simulink model "x64_32f_024i_gbe"  (Simulink 7.4) (continued)
== system x64_32f_024i_gbe == (continued)
  gpio_a_oen : xps_library/gpio  Tag=xps:gpio  SystemSampleTime=-1  io_group=ROACH:gpioa_oe_n  io_dir=out  arith_type=Boolean  bitwidth=1  bin_pt=0  bit_index=0  sample_period=1  use_single_ended=off  use_ddr=off  reg_iob=on  reg_clk_phase=0  termination=Pulldown
  led1 : xps_library/gpio  Tag=xps:gpio  SystemSampleTime=-1  io_group=ROACH:gpiob  io_dir=out  arith_type=Boolean  bitwidth=1  bin_pt=0  bit_index=1  sample_period=1  use_single_ended=off  use_ddr=off  reg_iob=on  reg_clk_phase=0  termination=Pulldown
  led2 : xps_library/gpio  Tag=xps:gpio  SystemSampleTime=-1  io_group=ROACH:gpiob  io_dir=out  arith_type=Boolean  bitwidth=1  bin_pt=0  bit_index=2  sample_period=1  use_single_ended=off  use_ddr=off  reg_iob=on  reg_clk_phase=0  termination=Pulldown
  led3 : xps_library/gpio  Tag=xps:gpio  SystemSampleTime=-1  io_group=ROACH:gpiob  io_dir=out  arith_type=Boolean  bitwidth=1  bin_pt=0  bit_index=3  sample_period=1  use_single_ended=off  use_ddr=off  reg_iob=on  reg_clk_phase=0  termination=Pulldown
  negedge : casper_library_misc/negedge  Tag=Rising Edge Detector  SystemSampleTime=-1
  never{,1..3,5..6,10} (7 alike) : xbsIndex_r4/Constant  arith_type=Boolean  const=0  n_bits=16  bin_pt=14  explicit_period=on  period=1  equ=P=C  opselect=C  inp2=PCIN>>17  opr=+  inp1=P  carry=CIN  dbl_ovrd=off  block_type=constant
      settings: block_version=11.4
  never4 : xbsIndex_r4/Constant  arith_type=Unsigned  const=0  n_bits=16  bin_pt=0  explicit_period=on  period=1  equ=P=C  opselect=C  inp2=PCIN>>17  opr=+  inp1=P  carry=CIN  dbl_ovrd=off  block_type=constant
      settings: block_version=11.4
  never8 : xbsIndex_r4/Constant  arith_type=Unsigned  const=0  n_bits=32  bin_pt=0  explicit_period=on  period=1  equ=P=C  opselect=C  inp2=PCIN>>17  opr=+  inp1=P  carry=CIN  dbl_ovrd=off  block_type=constant
      settings: block_version=11.4
  not_zero : Subsystem
  pfb_fir_mux1 : Subsystem  AncestorBlock=casper_library_pfbs/pfb_fir_mux  mask(pfb_fir_mux){PFBSize=11, mux_inputs=2, TotalTaps=4, WindowType=hamming, n_inputs=0, MakeBiplex=1, BitWidthIn=12, BitWidthOut=18, CoeffBitWidth=18, CoeffDistMem=0, add_latency=1, mult_latency=2, bram_latency=3, quantization=Round  (unbiased: +/- Inf), fwidth=1, specify_mult=off, mult_spec=[2                                      2]}
      mask prompts: PFBSize "Size of PFB: (2^? pnts)"; mux_inputs "Signals per Input (2^?)"; TotalTaps "Total Number of Taps:"; WindowType "Windowing Function:"; n_inputs "Number of Simultaneous Inputs: (2^?)"; MakeBiplex "Make Biplex"; BitWidthIn "Input Bitwidth:"; BitWidthOut "Output Bitwidth:"; CoeffBitWidth "Coefficient Bitwidth:"; CoeffDistMem "Use Distributed Memory for Coeffs"; add_latency "Add Latency"; mult_latency "Mult Latency"; bram_latency "BRAM Latency"; quantization "Quantization Behavior"; fwidth "Bin Width Scaling (normal=1)"; specify_mult "Specify multiplier use (behavioral if not specified)"; mult_spec "Multiplier specification (0=core, 1=embedded, 2=behavioural) (left=1st tap)"
      mask init:
      | pfb_fir_mux_init(gcb, ...
      |     'PFBSize', PFBSize, ...
      |     'mux_inputs', mux_inputs, ...
      |     'TotalTaps', TotalTaps, ...
      |     'WindowType', WindowType, ...
      |     'n_inputs', n_inputs, ...
      |     'MakeBiplex', MakeBiplex, ...
      |     'BitWidthIn', BitWidthIn, ...
      |     'BitWidthOut', BitWidthOut, ...
      |     'CoeffBitWidth', CoeffBitWidth, ...
      |     'CoeffDistMem', CoeffDistMem, ...
      |     'add_latency', add_latency, ...
      |     'mult_latency', mult_latency, ...
      |     'bram_latency', bram_latency, ...
      |     'quantization', quantization, ...
      |     'fwidth', fwidth,...
      |     'specify_mult', specify_mult,...
      |     'mult_spec', mult_spec);
      settings: AttributesFormatString=taps=4, add_latency=1
      mask Help: eval('xlWeb(''http://casper.berkeley.edu/wiki/Pfb_fir'')')
      mask StyleString: edit,edit,edit,popup(bartlett|barthannwin|blackman|blackmanharris|bohamwin|chebwin|flattopwin|gausswin|hamming|hann|kaiser|nuttallwin|parzenwin|rectwin|tukeywin|triang|userwindow),edit,edit,edit,edit,edit,edit,edit,edit,edit,popup(Truncate|Round  (unbiased: +/- Inf)|Round  (unbiased: Even Values)),edit,checkbox,edit
      mask CallbackString:
      | ||%num_taps = eval(get_param(gcb, 'TotalTaps'));
      | %old_vec = eval(get_param(gcb, 'mult_spec'));
      | 
      | %vec = 2.*ones(1,num_taps);
      | %old_size = length(old_vec);
      | 
      | %if(old_size <= num_taps),
      | %   new_size = old_size;
      | %else,
      | %    new_size = num_taps;
      | %end
      | 
      | %for n = 1:new_size, vec(n) = old_vec(n); end
      | %set_param(gcb, 'mult_spec', mat2str(vec));||||||||||||%num_taps = eval(get_param(gcb, 'TotalTaps'));
      | %old_vec = eval(get_param(gcb, 'mult_spec'));
      | 
      | %vec = 2.*ones(1,num_taps);
      | %old_size = length(old_vec);
      | 
      | %if(old_size <= num_taps),
      | %    new_size = old_size;
      | %else,
      | %    new_size = num_taps;
      | %end
      | 
      | %for n = 1:new_size, vec(n) = old_vec(n); end
      | %set_param(gcb, 'mult_spec', mat2str(vec));|%specify_mult = get_param(gcb,'specify_mult');
      | %if( strcmp(specify_mult,'off')),
      | %    set_param(gcb, 'MaskEnables', {'on','on','on','on','on','on','on','on','on','on','on','on','on','on','on','off'});
      | %else
      | %    set_param(gcb, 'MaskEnables', {'on','on','on','on','on','on','on','on','on','on','on','on','on','on','on','on'});
      | %end
      | 
      | |%specify_mult = get_param(gcb,'specify_mult');
      | %if( strcmp(specify_mult,'off')),
      | %    set_param(gcb, 'MaskEnables', {'on','on','on','on','on','on','on','on','on','on','on','on','on','on','on','off'});
      | %else
      | %    set_param(gcb, 'MaskEnables', {'on','on','on','on','on','on','on','on','on','on','on','on','on','on','on','on'});
      | %end
  pfb_fir_mux2 : Subsystem  AncestorBlock=casper_library_pfbs/pfb_fir_mux  mask(pfb_fir_mux){PFBSize=11, mux_inputs=2, TotalTaps=4, WindowType=hamming, n_inputs=0, MakeBiplex=1, BitWidthIn=12, BitWidthOut=18, CoeffBitWidth=18, CoeffDistMem=0, add_latency=1, mult_latency=2, bram_latency=3, quantization=Round  (unbiased: +/- Inf), fwidth=1, specify_mult=off, mult_spec=[2                                      2]}
      mask prompts: PFBSize "Size of PFB: (2^? pnts)"; mux_inputs "Signals per Input (2^?)"; TotalTaps "Total Number of Taps:"; WindowType "Windowing Function:"; n_inputs "Number of Simultaneous Inputs: (2^?)"; MakeBiplex "Make Biplex"; BitWidthIn "Input Bitwidth:"; BitWidthOut "Output Bitwidth:"; CoeffBitWidth "Coefficient Bitwidth:"; CoeffDistMem "Use Distributed Memory for Coeffs"; add_latency "Add Latency"; mult_latency "Mult Latency"; bram_latency "BRAM Latency"; quantization "Quantization Behavior"; fwidth "Bin Width Scaling (normal=1)"; specify_mult "Specify multiplier use (behavioral if not specified)"; mult_spec "Multiplier specification (0=core, 1=embedded, 2=behavioural) (left=1st tap)"
      mask init: same as x64_32f_024i_gbe/pfb_fir_mux1
      settings: AttributesFormatString=taps=4, add_latency=1
      mask Help: eval('xlWeb(''http://casper.berkeley.edu/wiki/Pfb_fir'')')
      mask StyleString: edit,edit,edit,popup(bartlett|barthannwin|blackman|blackmanharris|bohamwin|chebwin|flattopwin|gausswin|hamming|hann|kaiser|nuttallwin|parzenwin|rectwin|tukeywin|triang|userwindow),edit,edit,edit,edit,edit,edit,edit,edit,edit,popup(Truncate|Round  (unbiased: +/- Inf)|Round  (unbiased: Even Values)),edit,checkbox,edit
      mask CallbackString:
      | ||%num_taps = eval(get_param(gcb, 'TotalTaps'));
      | %old_vec = eval(get_param(gcb, 'mult_spec'));
      | 
      | %vec = 2.*ones(1,num_taps);
      | %old_size = length(old_vec);
      | 
      | %if(old_size <= num_taps),
      | %   new_size = old_size;
      | %else,
      | %    new_size = num_taps;
      | %end
      | 
      | %for n = 1:new_size, vec(n) = old_vec(n); end
      | %set_param(gcb, 'mult_spec', mat2str(vec));||||||||||||%num_taps = eval(get_param(gcb, 'TotalTaps'));
      | %old_vec = eval(get_param(gcb, 'mult_spec'));
      | 
      | %vec = 2.*ones(1,num_taps);
      | %old_size = length(old_vec);
      | 
      | %if(old_size <= num_taps),
      | %    new_size = old_size;
      | %else,
      | %    new_size = num_taps;
      | %end
      | 
      | %for n = 1:new_size, vec(n) = old_vec(n); end
      | %set_param(gcb, 'mult_spec', mat2str(vec));|%specify_mult = get_param(gcb,'specify_mult');
      | %if( strcmp(specify_mult,'off')),
      | %    set_param(gcb, 'MaskEnables', {'on','on','on','on','on','on','on','on','on','on','on','on','on','on','on','off'});
      | %else
      | %    set_param(gcb, 'MaskEnables', {'on','on','on','on','on','on','on','on','on','on','on','on','on','on','on','on'});
      | %end
      | 
      | |%specify_mult = get_param(gcb,'specify_mult');
      | %if( strcmp(specify_mult,'off')),
      | %    set_param(gcb, 'MaskEnables', {'on','on','on','on','on','on','on','on','on','on','on','on','on','on','on','off'});
      | %else
      | %    set_param(gcb, 'MaskEnables', {'on','on','on','on','on','on','on','on','on','on','on','on','on','on','on','on'});
      | %end
  phase_EQ0 : Subsystem  mask{N=11-1+5-2-1, bram_lat=2, mult_lat=3, add_lat=2}
      mask prompts: N "number of coefficients (2^?)"; bram_lat "bram latency"; mult_lat "multiplier latency"; add_lat "adder latency"
      mask init:
      | set_param([gcb, '/coeff_bram'], 'addr_width', num2str(N));
  phase_EQ1 : Subsystem  mask{N=11-1+5-2-1, bram_lat=2, mult_lat=3, add_lat=2}
      mask prompts: N "number of coefficients (2^?)"; bram_lat "bram latency"; mult_lat "multiplier latency"; add_lat "adder latency"
      mask init:
      | set_param([gcb, '/coeff_bram'], 'addr_width', num2str(N));
  phase_EQ2 : Subsystem  mask{N=11-1+5-2-1, bram_lat=2, mult_lat=3, add_lat=2}
      mask prompts: N "number of coefficients (2^?)"; bram_lat "bram latency"; mult_lat "multiplier latency"; add_lat "adder latency"
      mask init:
      | set_param([gcb, '/coeff_bram'], 'addr_width', num2str(N));
  phase_EQ3 : Subsystem  mask{N=11-1+5-2-1, bram_lat=2, mult_lat=3, add_lat=2}
      mask prompts: N "number of coefficients (2^?)"; bram_lat "bram latency"; mult_lat "multiplier latency"; add_lat "adder latency"
      mask init:
      | set_param([gcb, '/coeff_bram'], 'addr_width', num2str(N));
  pipeline : Subsystem  AncestorBlock=casper_library/Delays/pipeline  mask(pipeline){latency=1}
      mask help:
      | An explicitly laid-out delay line to avoid mapping to SRL16s
      | for use in pipelining to help achieve timing closure.
      mask init:
      | pipeline_init(gcb, 'latency', latency);
      mask Display:
      | port_label('input', 1, 'd');
      | port_label('output', 1, 'q');
      | disp(['Z^-', num2str(latency)]);
  pipeline102 : Subsystem  AncestorBlock=casper_library/Delays/pipeline  mask(pipeline){latency=1}
      mask help:
      | An explicitly laid-out delay line to avoid mapping to SRL16s
      | for use in pipelining to help achieve timing closure.
      mask init:
      | pipeline_init(gcb, 'latency', latency);
      mask Display:
      | port_label('input', 1, 'd');
      | port_label('output', 1, 'q');
      | disp(['Z^-', num2str(latency)]);
  pipeline3 : Subsystem  AncestorBlock=casper_library/Delays/pipeline  mask(pipeline){latency=1}
      mask help:
      | An explicitly laid-out delay line to avoid mapping to SRL16s
      | for use in pipelining to help achieve timing closure.
      mask init:
      | pipeline_init(gcb, 'latency', latency);
      mask Display:
      | port_label('input', 1, 'd');
      | port_label('output', 1, 'q');
      | disp(['Z^-', num2str(latency)]);
  qdr_transpose1 : Subsystem  AncestorBlock=casper_library/Reorder/qdr_transpose  mask{idepth=(11-1), odepth=10, which_qdr=qdr1}
      mask prompts: idepth "Input blocksize (2^n)"; odepth "Output blocksize (2^n)"; which_qdr "QDR Chip"
      mask init:
      | new_map = tostring([[0:2^odepth-1]*2 [0:2^odepth-1]*2 + 1]);
      | set_param([gcb, '/reorder'], 'map', new_map);
      | set_param([gcb, '/qdr'], 'which_qdr', which_qdr);
      mask StyleString: edit,edit,popup(qdr0|qdr1)
  quant : Subsystem
  quant1 : Subsystem
  quant2 : Subsystem
  quant3 : Subsystem
  quant4 : Subsystem
  quant5 : Subsystem
  quant6 : Subsystem
  quant7 : Subsystem
  reorder : Subsystem  AncestorBlock=casper_library/Reorder/reorder  mask(reorder){map=getfield( getfield( get_param( gcb,'UserData' ), 'parameters'),'map'), n_inputs=1, bram_latency=2, map_latency=0, double_buffer=0, bram_map=off}
      mask prompts: map "Output Order:"; n_inputs "Number of inputs"; bram_latency "BRAM Latency"; map_latency "Map Latency"; double_buffer "Double Buffer"; bram_map "Use BlockRAM for Map"
      mask init:
      | reorder_init(gcb, ...
      |     'map', map, ...
      |     'n_inputs', n_inputs, ...
      |     'bram_latency', bram_latency, ...
      |     'map_latency', map_latency, ...
      |     'double_buffer', double_buffer, ...
      |     'bram_map', bram_map);
      settings: AttributesFormatString=order=10
      mask Help: eval('xlWeb(''http://casper.berkeley.edu/wiki/Reorder'')')
  reorder_one_ant_a_time1 : Subsystem  mask{spec_chans=11-1, block_size=7}
      mask prompts: spec_chans "frequency channels? (2^?) (NFFT/2 for real FFTs)"; block_size "block size (2^?)"
      mask init:
      | partial_reorder = [0:2:2^block_size - 1]
      | 
      | reorder = []
      | for n = [0:1]
      |     reorder = [reorder, [partial_reorder]+n];
      | end
      | 
      | reorder
      | 
      | set_param([gcb, '/reorder'], 'map', tostring(reorder));
  revision_control : Subsystem  AncestorBlock=casper_library_misc/revision_control  mask(revision_control){rev_major=24, rev_minor=1, error_on_dirty=off, error_on_norcs=off}
      mask prompts: rev_major "Major revision number"; rev_minor "Minor revision number"; error_on_dirty "Error on out-of-date"; error_on_norcs "Error on no revision control"
      mask init:
      | revision_control_init( gcb, ...
      |   'rev_major', rev_major, ...
      |   'rev_minor', rev_minor, ...
      |   'error_on_dirty', error_on_dirty, ...
      |   'error_on_norcs', error_on_norcs);
  ri_to_c{,1..3} (4 alike) : casper_library_misc/ri_to_c  SystemSampleTime=-1
  seng_oob_signal : Subsystem
  seng_packetizer : Subsystem  mask{nwrd_bits=5, ant_bits=4}
      mask prompts: nwrd_bits "Payload length: (in 64bit words) (2^n)"; ant_bits "Number of windows per channel(2^?)"
      mask help: Package 32 bit data into 64 bits and identify data as coming from antenna 0 or 1
  sim_sync : ox_lib/sim_sync  SystemSampleTime=-1  delay=9
  sim_sync1 : ox_lib/sim_sync  SystemSampleTime=-1  delay=10
  snap_ctrl : xps_library/software register  Tag=xps:sw_reg  SystemSampleTime=-1  io_dir=From Processor  arith_type=Unsigned  bitwidth=32  bin_pt=0  sample_period=1  gw_name=x64_32f_024i_gbe_snap_ctrl_user_data_out
  status : Subsystem  Tag=xps:sw_reg  mask{io_dir=To Processor, arith_type=Unsigned, bitwidth=32, bin_pt=0, sample_period=1, latency=0}
      mask prompts: io_dir "I/O direction"; arith_type "Data Type"; bitwidth "Data bitwidth"; bin_pt "Data binary point"; sample_period "Sample period"
      mask init:
      | gw_name = clear_name(gcb);
      | config.source = str2func('software_register_init');
      | config.toplevel = gcb;
      | xBlock( config, {io_dir, arith_type, bitwidth, bin_pt, ... 
      |     sample_period, gw_name, latency});
      mask StyleString: popup(From Processor|To Processor),popup(Unsigned|Signed  (2's comp)),edit,edit,edit,edit
  sync_gen : Subsystem  mask{period=10010*2^20}
  sync_gpio : xps_library/gpio  Tag=xps:gpio  SystemSampleTime=-1  io_group=ROACH:gpioa  io_dir=in  arith_type=Boolean  bitwidth=1  bin_pt=0  bit_index=7  sample_period=1  use_single_ended=off  use_ddr=off  reg_iob=on  reg_clk_phase=0  termination=Pullup
  sync_rate_change : Subsystem  mask{bits=10+3}
      mask prompts: bits "New sync rate: 2^? clocks?"
      mask help: Increases the sync pulses. For use in, for example, a snap block, where the snap can be triggered every 2^NFFT clocks, but the system sync may come much less frequently.
  sync_rate_change1 : Subsystem  mask{bits=10+3}
      mask prompts: bits "New sync rate: 2^? clocks?"
      mask help: Increases the sync pulses. For use in, for example, a snap block, where the snap can be triggered every 2^NFFT clocks, but the system sync may come much less frequently.
  sync_rate_change2 : Subsystem  mask{bits=10+3}
      mask prompts: bits "New sync rate: 2^? clocks?"
      mask help: Increases the sync pulses. For use in, for example, a snap block, where the snap can be triggered every 2^NFFT clocks, but the system sync may come much less frequently.
  sync_rate_change3 : Subsystem  mask{bits=10+3}
      mask prompts: bits "New sync rate: 2^? clocks?"
      mask help: Increases the sync pulses. For use in, for example, a snap block, where the snap can be triggered every 2^NFFT clocks, but the system sync may come much less frequently.
  sync_rate_change4 : Subsystem  mask{bits=10+3}
      mask prompts: bits "New sync rate: 2^? clocks?"
      mask help: Increases the sync pulses. For use in, for example, a snap block, where the snap can be triggered every 2^NFFT clocks, but the system sync may come much less frequently.
  ten_Gbe_v2 : xps_library/ten_Gbe_v2  Tag=xps:tengbe_v2  SystemSampleTime=-1  flavour=cx4  slot=1  port_r1=2  port_r2_cx4=0  port_r2_sfpp=0  rx_dist_ram=on  large_frames=on  show_param=on  pre_emph_r2=0.74  pre_emph=3  post_emph_r2=0.18  rxeqmix_r2=7  swing_r2=940  swing=800  fab_en=on  fab_mac=hex2dec('123456780000')  fab_ip=192*(2^24) + 168*(2^16) + 5*(2^8) + 20*(2^0)  fab_udp=10000  fab_gate=1  cpu_rx_en=on  cpu_tx_en=on
  uncram : Subsystem  AncestorBlock=gavrt_library/uncram  mask(uncram){num_slice=8, slice_width=1, bin_pt=0, arith_type=0}
      mask prompts: num_slice "Number of slices"; slice_width "Slice Width"; bin_pt "Output Binary Point"; arith_type "Output Arithmetic Type (0=Unsigned, 1=Signed)"
      mask help: Takes a concatenated input and slices it up into even pieces and reinterprets them as signed fixed point numbers with a given binary point.
      mask init:
      | uncram_init(gcb, ...
      |     'num_slice', num_slice, ...
      |     'slice_width', slice_width, ...
      |     'bin_pt', bin_pt, ...
      |     'arith_type', arith_type);
      settings: AttributesFormatString=UFix_1_0
  uncram1 : Subsystem  AncestorBlock=gavrt_library/uncram  mask(uncram){num_slice=8, slice_width=1, bin_pt=0, arith_type=0}
      mask prompts: num_slice "Number of slices"; slice_width "Slice Width"; bin_pt "Output Binary Point"; arith_type "Output Arithmetic Type (0=Unsigned, 1=Signed)"
      mask help: Takes a concatenated input and slices it up into even pieces and reinterprets them as signed fixed point numbers with a given binary point.
      mask init:
      | uncram_init(gcb, ...
      |     'num_slice', num_slice, ...
      |     'slice_width', slice_width, ...
      |     'bin_pt', bin_pt, ...
      |     'arith_type', arith_type);
      settings: AttributesFormatString=UFix_1_0
  x64_adc : Subsystem  Tag=xps:x64_adc  AncestorBlock=xps_library/x64_adc  mask(x64_adc){adc_clk_rate=40, spi=off, ctrl_gpio=GPIO_B}
      mask prompts: adc_clk_rate "ADC clock rate (MHz)"; spi "Include SPI interface?"; ctrl_gpio "GPIO bank to use for control signals?"
      mask help: The ADC block converts analog inputs to digital outputs. Every clock cycle, the inputs are sampled and digitized to 8 bit binary point numbers in the range of [-1, 1) and are then output by the adc.
      mask init:
      | x64_adc_mask(gcb, 'spi', spi);
      mask Help: eval('xlWeb([getenv(''MLIB_ROOT''), ''\doc\html\sys_adc\sys_adc.html''])')
      mask StyleString: edit,checkbox,popup(GPIO_A|GPIO_B)
  x_snap : Subsystem
  xeng_oob_signal : Subsystem
  xeng_packetiser : Subsystem  mask{nwrd_bits=5, ant_bits=4}
      mask prompts: nwrd_bits "Payload length: (in 64bit words) (2^n)"; ant_bits "Number of antennas on this link (2^n)"
      mask help: Package 32 bit data into 64 bits and identify data as coming from antenna 0 or 1
  xengine_mcnt_lsb : Subsystem  Tag=xps:sw_reg  mask{io_dir=To Processor, arith_type=Unsigned, bitwidth=32, bin_pt=0, sample_period=1, latency=0}
      mask prompts: io_dir "I/O direction"; arith_type "Data Type"; bitwidth "Data bitwidth"; bin_pt "Data binary point"; sample_period "Sample period"
      mask init:
      | gw_name = clear_name(gcb);
      | config.source = str2func('software_register_init');
      | config.toplevel = gcb;
      | xBlock( config, {io_dir, arith_type, bitwidth, bin_pt, ... 
      |     sample_period, gw_name, latency});
      mask StyleString: popup(From Processor|To Processor),popup(Unsigned|Signed  (2's comp)),edit,edit,edit,edit
  xengine_mcnt_msb : Subsystem  Tag=xps:sw_reg  mask{io_dir=To Processor, arith_type=Unsigned, bitwidth=32, bin_pt=0, sample_period=1, latency=0}
      mask prompts: io_dir "I/O direction"; arith_type "Data Type"; bitwidth "Data bitwidth"; bin_pt "Data binary point"; sample_period "Sample period"
      mask init:
      | gw_name = clear_name(gcb);
      | config.source = str2func('software_register_init');
      | config.toplevel = gcb;
      | xBlock( config, {io_dir, arith_type, bitwidth, bin_pt, ... 
      |     sample_period, gw_name, latency});
      mask StyleString: popup(From Processor|To Processor),popup(Unsigned|Signed  (2's comp)),edit,edit,edit,edit
  wires:
  adc_spi_ctrl/1 -> Slice3/1
  Mux1/1 -> [adc0_3_in]
  Mux2/1 -> [adc4_7_in]
  Mux3/1 -> [adc8_11_in]
  Mux4/1 -> [adc12_15_in]
  Mux5/1 -> [adc16_19_in]
  Mux6/1 -> [adc20_23_in]
  Mux7/1 -> [adc24_27_in]
  Mux8/1 -> [adc28_31in]
  Delay8/1 -> Delay4/1, [adc_chan_sync0]
  [debug_sigs] -> Mux1/1, Mux2/1, Mux3/1, Mux4/1, Mux5/1, Mux6/1, Mux7/1, Mux8/1
  x64_adc/17(chan_sync) -> Delay8/1, Delay6/1
  x64_adc/1(dout0) -> Mux1/2
  x64_adc/3(dout2) -> Mux2/2
  x64_adc/5(dout4) -> Mux3/2
  x64_adc/7(dout6) -> Mux4/2
  x64_adc/9(dout8) -> Mux5/2
  x64_adc/11(dout10) -> Mux6/2
  x64_adc/13(dout12) -> Mux7/2
  x64_adc/15(dout14) -> Mux8/2
  [adc0_3_in] -> adc_sw/1(in0)
  [adc4_7_in] -> adc_sw/2(in1)
  [adc8_11_in] -> adc_sw/3(in2)
  [adc12_15_in] -> adc_sw/4(in3)
  [adc16_19_in] -> adc_sw/5(in4)
  [adc20_23_in] -> adc_sw/6(in5)
  [adc24_27_in] -> adc_sw/7(in6)
  [adc28_31in] -> adc_sw/8(in7)
  [adc_chan_sync0] -> Slice1/1
  Slice1/1 -> adc_sw/9(chan_sync)
  [adc_chan_sync] -> uncram/1(In)
  uncram/1(Out1) -> Logical1/1
  uncram/2(Out2) -> Logical1/2
  uncram/3(Out3) -> Logical1/3
  uncram/4(Out4) -> Logical1/4
  uncram/5(Out5) -> Logical1/5
  uncram/6(Out6) -> Logical1/6
  uncram/7(Out7) -> Logical1/7
  uncram/8(Out8) -> Logical1/8
  Logical1/1 -> Convert/1
  [sync_gen] -> Delay/1
  Delay/1 -> Logical2/1
  [adc_chan_sync] -> Slice9/1
  Slice9/1 -> Inverter/1
  Inverter/1 -> Logical2/2
  Logical2/1 -> Convert1/1
  ext22/1(out) -> Concat6/1, Logical8/1
  ext1/1(out) -> Concat6/2, Logical8/2
  Concat6/1 -> adc_sync_test/1(reg_out)
  Convert/1 -> ext22/1(in)
  Convert1/1 -> ext1/1(in)
  Constant20/1 -> ctrl_sw/1
  ctrl_sw/1 -> Slice/1, Slice4/1, Slice2/1, Slice12/1, Slice11/1, Slice14/1, Slice15/1, Slice22/1, Slice16/1
  Slice4/1 -> [arm_rst]
  Slice/1 -> [shift]
  Slice2/1 -> [sync_rst]
  sync_gen/1(sync) -> sim_sync1/1
  [arm_rst] -> sync_gen/2(arm)
  sync_gen/3(armed) -> [armed]
  Logical7/1 -> Convert11/1
  sync_gpio/1 -> negedge/1
  [sync_rst] -> Logical7/2
  sim_sync1/1 -> adc_sync_align/1(sync)
  adc_sync_align/1(sync_out) -> [sync_gen]
  sync_gen/2(mrst) -> sim_sync/1
  adc_sync_align1/1(sync_out) -> [mrst]
  [adc_chan_sync] -> Slice7/1
  Slice12/1 -> [xaui_rcv_rst]
  Slice7/1 -> adc_sync_align/2(adc_sync), adc_sync_align1/2(adc_sync)
  Delay3/1 -> adc_sync_align1/1(sync)
  sim_sync/1 -> Delay3/1
  Slice11/1 -> [debug_sigs]
  [fft_sync_out] -> amp_EQ0/1(sync_in)
  [fft0_7_out] -> amp_EQ0/2(din)
  [fft_sync_out] -> amp_EQ1/1(sync_in)
  [fft8_15_out] -> amp_EQ1/2(din)
  amp_EQ0/1(dout) -> [amp_EQ_0_7_out]
  amp_EQ0/2(sync_out) -> [amp_EQ_sync_out]
  amp_EQ1/1(dout) -> [amp_EQ_8_15_out]
  [fft_sync_out] -> amp_EQ2/1(sync_in)
  [fft16_23_out] -> amp_EQ2/2(din)
  amp_EQ2/1(dout) -> [amp_EQ_16_23_out]
  [fft_sync_out] -> amp_EQ3/1(sync_in)
  [fft24_31_out] -> amp_EQ3/2(din)
  amp_EQ3/1(dout) -> [amp_EQ_24_31_out]
  amp_EQ0/3(of) -> [amp_of_0]
  amp_EQ1/3(of) -> [amp_of_1]
  amp_EQ2/3(of) -> [amp_of_2]
  amp_EQ3/3(of) -> [amp_of_3]
  phase_EQ0/1(dout) -> [phase_EQ_0_7_out]
  phase_EQ0/2(sync_out) -> [phase_EQ_sync_out]
  phase_EQ1/1(dout) -> [phase_EQ_8_15_out]
  phase_EQ2/1(dout) -> [phase_EQ_16_23_out]
  phase_EQ3/1(dout) -> [phase_EQ_24_31_out]
  [amp_EQ_sync_out] -> phase_EQ0/1(sync_in)
  [amp_EQ_0_7_out] -> phase_EQ0/2(din)
  [amp_EQ_sync_out] -> phase_EQ1/1(sync_in)
  [amp_EQ_8_15_out] -> phase_EQ1/2(din)
  [amp_EQ_sync_out] -> phase_EQ2/1(sync_in)
  [amp_EQ_16_23_out] -> phase_EQ2/2(din)
  [amp_EQ_sync_out] -> phase_EQ3/1(sync_in)
  [amp_EQ_24_31_out] -> phase_EQ3/2(din)
  Concat4/1 -> [quant_fftt]
  Concat3/1 -> [quant_x_eng]
  [amp_EQ_0_7_out] -> quant/1(din)
  [amp_EQ_8_15_out] -> quant1/1(din)
  [amp_EQ_16_23_out] -> quant2/1(din)
  [amp_EQ_24_31_out] -> quant3/1(din)
  [phase_EQ_0_7_out] -> quant4/1(din)
  [phase_EQ_8_15_out] -> quant5/1(din)
  [phase_EQ_16_23_out] -> quant6/1(din)
  [phase_EQ_24_31_out] -> quant7/1(din)
  quant4/1(dout) -> Concat4/1
  quant5/1(dout) -> Concat4/2
  quant6/1(dout) -> Concat4/3
  quant7/1(dout) -> Concat4/4
  quant/1(dout) -> Concat3/1
  quant1/1(dout) -> Concat3/2
  quant2/1(dout) -> Concat3/3
  quant3/1(dout) -> Concat3/4
  [amp_EQ_sync_out] -> Delay1/1
  [phase_EQ_sync_out] -> Delay2/1
  Delay2/1 -> [quant_fftt_sync]
  Delay1/1 -> [quant_x_eng_sync]
  quant4/2(of) -> [quant_of_f0]
  quant5/2(of) -> [quant_of_f1]
  quant6/2(of) -> [quant_of_f2]
  quant7/2(of) -> [quant_of_f3]
  quant/2(of) -> [quant_of_x0]
  quant1/2(of) -> [quant_of_x1]
  quant2/2(of) -> [quant_of_x2]
  quant3/2(of) -> [quant_of_x3]
  [quant_fftt] -> fftt_transpose/2(d)
  [quant_fftt_sync] -> fftt_transpose/1(sync)
  fftt_transpose/2(q) -> Slice8/1
  fftt_transpose/1(sync_out) -> [reorder_fftt_sync_out]
  Slice8/1 -> [fftt_reorder_out]
  Constant/1 -> reorder/2(en)
  qdr_transpose1/1(sync_out) -> reorder/1(sync)
  qdr_transpose1/2(q) -> Slice13/1
  reorder/1(sync_out) -> reorder_one_ant_a_time1/1(sync_in)
  reorder/3(dout0) -> reorder_one_ant_a_time1/2(din)
  reorder_one_ant_a_time1/1(sync_out) -> [reorder_X_sync_out], bit_use_mon/1(sync)
  reorder_one_ant_a_time1/3(dout) -> [X_reorder_out], bit_use_mon/2(din)
  Slice13/1 -> reorder/3(din0)
  seng_oob_signal/1(data_out) -> [fftt_xaui64]
  seng_oob_signal/2(oob) -> [oob_fftt]
  seng_oob_signal/3(valid_out) -> [tx_en_fftt]
  xeng_packetiser/1(data_out) -> xeng_oob_signal/1(data_in)
  xeng_packetiser/2(valid_out) -> xeng_oob_signal/2(valid_in)
  xeng_packetiser/3(header_out) -> xeng_oob_signal/3(header)
  xeng_packetiser/4(eof) -> xeng_oob_signal/4(eof), [eof]
  xeng_packetiser/5(sync_out) -> xeng_oob_signal/5(sync)
  xeng_packetiser/6(mrst_out) -> xeng_oob_signal/6(mrst)
  [X_reorder_out] -> xeng_packetiser/1(data_in)
  [reorder_X_sync_out] -> xeng_packetiser/2(sync_in)
  [mrst] -> xeng_packetiser/3(mrst_in)
  xeng_oob_signal/1(data_out) -> [X_xaui64]
  xeng_oob_signal/3(valid_out) -> [tx_en_X]
  xeng_oob_signal/2(oob) -> [X_xaui_oob]
  seng_packetizer/1(data_out) -> seng_oob_signal/1(data_in)
  seng_packetizer/2(valid_out) -> seng_oob_signal/2(valid_in)
  seng_packetizer/3(header_out) -> seng_oob_signal/3(header)
  seng_packetizer/4(eof) -> seng_oob_signal/4(eof)
  seng_packetizer/5(sync_out) -> seng_oob_signal/5(sync)
  seng_packetizer/6(mrst_out) -> seng_oob_signal/6(mrst)
  [mrst] -> seng_packetizer/3(mrst_in)
  [reorder_fftt_sync_out] -> seng_packetizer/2(sync_in)
  [fftt_reorder_out] -> seng_packetizer/1(data_in)
  Constant7/1 -> XAUI_S/1
  [xaui_rcv_rst] -> XAUI_S/2
  [tx_en_fftt] -> XAUI_S/5
  [fftt_xaui64] -> XAUI_S/3
  [oob_fftt] -> XAUI_S/4
  Constant1/1 -> XAUI_X/1
  [xaui_rcv_rst] -> XAUI_X/2
  [tx_en_X] -> XAUI_X/5
  [X_xaui64] -> XAUI_X/3
  [X_xaui_oob] -> XAUI_X/4
  XAUI_S/6 -> [s_xaui_of]
  Slice5/1 -> xengine_mcnt_msb/1(reg_out)
  xeng_packetiser/7(mcnt_out) -> Slice5/1, Slice6/1
  Slice6/1 -> xengine_mcnt_lsb/1(reg_out)
  Slice14/1 -> [white_noise]
  Mux9/1 -> [adc0_3_in1]
  Gaussian Random Number Generator/1 -> Reinterpret7/1
  [white_noise] -> Mux9/1, Mux10/1, Mux12/1, Mux11/1, Mux16/1, Mux13/1, Mux15/1, Mux14/1
  [adc0_3_in] -> Mux9/2
  Mux10/1 -> [adc4_7_in1]
  Mux12/1 -> [adc8_11_in1]
  Mux11/1 -> [adc12_15_in1]
  Mux16/1 -> [adc16_19_in1]
  Mux13/1 -> [adc20_23_in1]
  Mux15/1 -> [adc24_27_in1]
  Mux14/1 -> [adc28_31in1]
  [adc4_7_in] -> Mux10/2
  [adc8_11_in] -> Mux12/2
  [adc12_15_in] -> Mux11/2
  [adc16_19_in] -> Mux16/2
  [adc20_23_in] -> Mux13/2
  [adc24_27_in] -> Mux15/2
  [adc28_31in] -> Mux14/2
  Gaussian Random Number Generator/2 -> Reinterpret6/1
  Gaussian Random Number Generator/3 -> Reinterpret5/1
  Gaussian Random Number Generator/4 -> Reinterpret4/1
  Gaussian Random Number Generator/5 -> Reinterpret3/1
  Gaussian Random Number Generator/6 -> Reinterpret2/1
  Gaussian Random Number Generator/7 -> Reinterpret1/1
  Gaussian Random Number Generator/8 -> Reinterpret/1
  [white_noise_rst] -> Gaussian Random Number Generator/1
  Slice15/1 -> [white_noise_rst]
  Convert2/1 -> Mux14/3
  Convert3/1 -> Mux15/3
  Convert4/1 -> Mux13/3
  Convert5/1 -> Mux16/3
  Convert6/1 -> Mux11/3
  Convert7/1 -> Mux12/3
  Convert8/1 -> Mux10/3
  Convert9/1 -> Mux9/3
  Reinterpret/1 -> Convert2/1
  Reinterpret1/1 -> Convert3/1
  Reinterpret2/1 -> Convert4/1
  Reinterpret3/1 -> Convert5/1
  Reinterpret4/1 -> Convert6/1
  Reinterpret5/1 -> Convert7/1
  Reinterpret6/1 -> Convert8/1
  Reinterpret7/1 -> Convert9/1
  Concat1/1 -> pipeline/1(d)
  [fft_of_0] -> Logical3/1
  [fft_of_1] -> Logical3/2
  [armed] -> pipeline3/1(d)
  never1/1 -> Concat1/6
  never/1 -> Concat1/8
  never2/1 -> Concat1/11
  Regster1/1 -> Concat1/10
  Regster2/1 -> Concat1/9
  Regster3/1 -> Concat1/7
  Logical5/1 -> Regster3/3, Regster3/1
  Logical3/1 -> Regster2/3, Regster2/1
  Logical4/1 -> Regster1/3, Regster1/1
  Regster8/1 -> Concat1/1
  never3/1 -> Concat1/2
  Regster6/1 -> Concat1/3
  Regster5/1 -> Concat1/4
  Regster4/1 -> Concat1/5
  never4/1 -> not_zero/1(In1), Regster8/1
  never5/1 -> Regster6/3, Regster6/1
  never6/1 -> Regster5/3, Regster5/1
  pipeline3/1(q) -> Regster4/3, Regster4/1
  [status_rst] -> Regster2/2, Regster1/2
  [status_rst] -> Regster3/2
  [status_rst] -> Regster8/2, Regster6/2, Regster5/2, Regster4/2
  pipeline/1(q) -> status/1(reg_out)
  not_zero/1(Out1) -> Regster8/3
  [amp_of_0] -> Logical4/1
  [amp_of_1] -> Logical4/2
  [amp_of_2] -> Logical4/3
  [amp_of_3] -> Logical4/4
  [quant_of_f0] -> Logical5/1
  [quant_of_f1] -> Logical5/2
  [quant_of_f2] -> Logical5/3
  [quant_of_f3] -> Logical5/4
  Constant13/1 -> snap_ctrl/1
  snap_ctrl/1 -> x_snap/22(ctrl_reg)
  [fft_sync_out] -> sync_rate_change/1(In1)
  [fft0_7_out] -> x_snap/1(fft0)
  [fft8_15_out] -> x_snap/2(fft1)
  [fft16_23_out] -> x_snap/3(fft2)
  [fft24_31_out] -> x_snap/4(fft3)
  [amp_EQ_sync_out] -> sync_rate_change1/1(In1)
  [amp_EQ_0_7_out] -> x_snap/5(amp0)
  [amp_EQ_8_15_out] -> x_snap/6(amp1)
  [amp_EQ_16_23_out] -> x_snap/7(amp2)
  [amp_EQ_24_31_out] -> x_snap/8(amp3)
  [phase_EQ_0_7_out] -> x_snap/9(phase0)
  [phase_EQ_8_15_out] -> x_snap/10(phase1)
  [phase_EQ_16_23_out] -> x_snap/11(phase2)
  [phase_EQ_24_31_out] -> x_snap/12(phase3)
  [phase_EQ_sync_out] -> sync_rate_change2/1(In1)
  [quant_fftt] -> x_snap/13(quant0)
  sync_rate_change/1(Out1) -> x_snap/16(fft_sync)
  sync_rate_change1/1(Out1) -> x_snap/17(amp_sync)
  sync_rate_change2/1(Out1) -> x_snap/18(phase_sync)
  sync_rate_change3/1(Out1) -> x_snap/19(quant_sync)
  [quant_fftt_sync] -> sync_rate_change3/1(In1)
  [quant_x_eng] -> x_snap/14(quant1)
  never8/1 -> x_snap/15(x_transpose)
  [quant_x_eng_sync] -> sync_rate_change4/1(In1)
  never10/1 -> x_snap/21(x_transpose_sync)
  pipeline102/1(q) -> [status_rst]
  Slice22/1 -> pipeline102/1(d)
  Delay4/1 -> [adc_chan_sync]
  [fir_sync_out] -> a/1(sync)
  [fir0_3_out] -> a/2(in0)
  [fir4_7_out] -> a/3(in1)
  [fir8_11_out] -> a/4(in2)
  [fir12_15_out] -> a/5(in3)
  [fir16_19_out] -> a/6(in4)
  [fir20_23_out] -> a/7(in5)
  [fir24_27_out] -> a/8(in6)
  [fir28_31_out] -> a/9(in7)
  a/1(sync_out) -> [mux_sync_out]
  a/2(Out0) -> [mux0_3_out]
  a/3(Out1) -> [mux4_7_out]
  a/4(Out2) -> [mux8_11_out]
  a/5(Out3) -> [mux12_15_out]
  a/6(Out4) -> [mux16_19_out]
  a/7(Out5) -> [mux20_23_out]
  a/8(Out6) -> [mux24_27_out]
  a/9(Out7) -> [mux28_31_out]
  chan_reorder/1(sync_out) -> qdr_transpose1/1(sync)
  chan_reorder/2(dout) -> qdr_transpose1/2(d)
  x64_adc/16(dout15) -> Register/1
  Register/1 -> Mux8/3, Mux7/3, Mux6/3, Mux5/3, Mux4/3, Mux3/3, Mux2/3, Mux1/3
  Slice10/1 -> Register/2
  Slice16/1 -> [xeng_phasecal_mux]
  Mux17/1 -> chan_reorder/1(sync)
  Mux18/1 -> chan_reorder/2(din)
  [quant_x_eng_sync] -> Mux17/2
  [quant_x_eng] -> Mux18/2
  [xeng_phasecal_mux] -> Mux17/1
  [xeng_phasecal_mux] -> Mux18/1
  [quant_fftt] -> Mux18/3
  [quant_fftt_sync] -> Mux17/3
  Delay6/1 -> Slice10/1
  [adc_chan_sync] -> uncram1/1(In)
  uncram1/1(Out1) -> Logical6/1
  uncram1/2(Out2) -> Logical6/2
  uncram1/3(Out3) -> Logical6/3
  uncram1/4(Out4) -> Logical6/4
  uncram1/5(Out5) -> Logical6/5
  uncram1/6(Out6) -> Logical6/6
  uncram1/7(Out7) -> Logical6/7
  uncram1/8(Out8) -> Logical6/8
  Logical6/1 -> Convert10/1
  ext2/1(out) -> Concat6/3, Inverter1/1
  Convert10/1 -> ext2/1(in)
  sync_rate_change4/1(Out1) -> x_snap/20(quant_sync1)
  [mux_sync_out] -> fft_biplex_real_2x/1(sync)
  [shift] -> fft_biplex_real_2x/2(shift)
  [mux0_3_out] -> fft_biplex_real_2x/3(pol1)
  [mux8_11_out] -> fft_biplex_real_2x/4(pol2)
  [mux4_7_out] -> fft_biplex_real_2x/5(pol3)
  [mux12_15_out] -> fft_biplex_real_2x/6(pol4)
  fft_biplex_real_2x/1(sync_out) -> [fft_sync_out]
  fft_biplex_real_2x/2(pol12_out) -> [fft0_7_out]
  fft_biplex_real_2x/3(pol34_out) -> [fft8_15_out]
  fft_biplex_real_2x/4(of) -> [fft_of_0]
  [mux_sync_out] -> fft_biplex_real_2x1/1(sync)
  [shift] -> fft_biplex_real_2x1/2(shift)
  [mux16_19_out] -> fft_biplex_real_2x1/3(pol1)
  [mux24_27_out] -> fft_biplex_real_2x1/4(pol2)
  [mux20_23_out] -> fft_biplex_real_2x1/5(pol3)
  [mux28_31_out] -> fft_biplex_real_2x1/6(pol4)
  fft_biplex_real_2x1/2(pol12_out) -> [fft16_23_out]
  fft_biplex_real_2x1/3(pol34_out) -> [fft24_31_out]
  fft_biplex_real_2x1/4(of) -> [fft_of_1]
  [sync_gen] -> pfb_fir_mux2/1(sync)
  [adc0_3_in1] -> ri_to_c/1
  [adc4_7_in1] -> ri_to_c/2
  ri_to_c/1 -> pfb_fir_mux2/2(pol1_in1)
  ri_to_c1/1 -> pfb_fir_mux2/3(pol2_in1)
  [adc8_11_in1] -> ri_to_c1/1
  [adc12_15_in1] -> ri_to_c1/2
  pfb_fir_mux2/1(sync_out) -> [fir_sync_out]
  pfb_fir_mux2/2(pol1_out1) -> c_to_ri/1
  pfb_fir_mux2/3(pol2_out1) -> c_to_ri1/1
  c_to_ri/1 -> [fir0_3_out]
  c_to_ri/2 -> [fir4_7_out]
  c_to_ri1/1 -> [fir8_11_out]
  c_to_ri1/2 -> [fir12_15_out]
  [sync_gen] -> pfb_fir_mux1/1(sync)
  ri_to_c2/1 -> pfb_fir_mux1/2(pol1_in1)
  ri_to_c3/1 -> pfb_fir_mux1/3(pol2_in1)
  pfb_fir_mux1/2(pol1_out1) -> c_to_ri2/1
  pfb_fir_mux1/3(pol2_out1) -> c_to_ri3/1
  [adc16_19_in1] -> ri_to_c2/1
  [adc20_23_in1] -> ri_to_c2/2
  [adc24_27_in1] -> ri_to_c3/1
  [adc28_31in1] -> ri_to_c3/2
  c_to_ri2/1 -> [fir16_19_out]
  c_to_ri2/2 -> [fir20_23_out]
  c_to_ri3/1 -> [fir24_27_out]
  c_to_ri3/2 -> [fir28_31_out]
  Slice3/1 -> x64_adc/18(adc_rst)
  adc_sim_sync/1(Out1) -> x64_adc/17(sim_sync)
  negedge/1 -> Logical7/1
  Constant2/1 -> gpio_a_oen/1
  [sync_rst_led] -> ext3/1(in)
  [adc_error_led] -> ext4/1(in)
  Inverter1/1 -> Logical8/3
  Logical8/1 -> [adc_error_led]
  ext3/1(out) -> led1/1
  ext4/1(out) -> led2/1
  [sync_gen] -> ext5/1(in)
  ext5/1(out) -> led3/1
  Convert11/1 -> sync_gen/1(rst), [sync_rst_led]
  Constant3/1 -> sync_gpio/1
  [xaui_rcv_rst] -> ten_Gbe_v2/1
  [X_xaui64] -> ten_Gbe_v2/2
  [tx_en_X] -> ten_Gbe_v2/3
  gbe_dest_ip/1 -> ten_Gbe_v2/4
  gbe_dest_port/1 -> ten_Gbe_v2/5
  ten_Gbe_v2/12 -> ten_Gbe_v2/8
  Constant4/1 -> ten_Gbe_v2/7
  [eof] -> ten_Gbe_v2/6
